simulink model "cddDcmApp"  (R2022b)
settings: Solver=FixedStepDiscrete  StartTime=0.0  StopTime=10.0  FixedStep=0.02  RelTol=1e-3
undefined symbols (not defined in the file or in its folder): CCPFaultType, DcChlType, HFAChlType, IcuChannelType, MtrRoutStaEnum, P_SignalPartType, P_Std_ReturnType, PowerChlType, SrsAdChlType, VGAChlType
(part 2 of 13)

== system cddDcmApp/DataServices_DID_4756_DID_4756_Front_Mosfet_Blower_FB ==
  DataServices_DID_4756_DID_4756_Front_Mosfet_Blower_FB_Voltage_sys : Subsystem

== system cddDcmApp/DataServices_DID_47F3_DID_47F3_AIU_Failure_Reasons_and ==
  DataServices_DID_47F3_DID_47F3_AIU_Failure_Reasons_and_Status_sys : Subsystem

== system cddDcmApp/DataServices_DID_47F4_DID_47F4_AQS_Air_Quality ==
  DataServices_DID_47F4_DID_47F4_AQS_Air_Quality_Level_sys : Subsystem

== system cddDcmApp/DataServices_DID_47F5_DID_47F5_TestRunCriteria_TRC ==
  DataServices_DID_47F5_DID_47F5_TestRunCriteria_TRC_Result_sys : Subsystem

== system cddDcmApp/DataServices_DID_47F6_DID_47F6_CCM_Lin1_Frame ==
  DataServices_DID_47F6_DID_47F6_CCM_Lin1_Frame_Status_sys : Subsystem

== system cddDcmApp/DataServices_DID_47F7_DID_47F7_CCM_Lin2_Frame ==
  DataServices_DID_47F7_DID_47F7_CCM_Lin2_Frame_Status_sys : Subsystem

== system cddDcmApp/DataServices_DID_47F8_DID_47F8_CCM_Lin3_Frame ==
  DataServices_DID_47F8_DID_47F8_CCM_Lin3_Frame_Status_sys : Subsystem

== system cddDcmApp/DataServices_DID_47F9_DID_47F9_Blower_Max_Level_Voltage ==
  DataServices_DID_47F9_DID_47F9_Blower_Max_Level_Voltage_Limit_sys : Subsystem

== system cddDcmApp/DataServices_DID_4AF7_DID_4AF7_1_DC_Motor_Drive_Request_Number ==
  DataServices_DID_4AF7_DID_4AF7_1_DC_Motor_Drive_Request_Number_FreezeCurrentState_sys : Subsystem
  DataServices_DID_4AF7_DID_4AF7_1_DC_Motor_Drive_Request_Number_ReadData_sys : Subsystem
  DataServices_DID_4AF7_DID_4AF7_1_DC_Motor_Drive_Request_Number_ReturnControlToECU_sys : Subsystem
  DataServices_DID_4AF7_DID_4AF7_1_DC_Motor_Drive_Request_Number_ShortTermAdjustment_sys : Subsystem

== system cddDcmApp/DataServices_DID_4AF7_DID_4AF7_2_DC_Motor_Drive_Request_Time ==
  DataServices_DID_4AF7_DID_4AF7_2_DC_Motor_Drive_Request_Time_FreezeCurrentState_sys : Subsystem
  DataServices_DID_4AF7_DID_4AF7_2_DC_Motor_Drive_Request_Time_ReadData_sys : Subsystem
  DataServices_DID_4AF7_DID_4AF7_2_DC_Motor_Drive_Request_Time_ReturnControlToECU_sys : Subsystem
  DataServices_DID_4AF7_DID_4AF7_2_DC_Motor_Drive_Request_Time_ShortTermAdjustment_sys : Subsystem

== system cddDcmApp/DataServices_DID_4AF7_DID_4AF7_3_DC_Motor_Drive_Request_Direction ==
  DataServices_DID_4AF7_DID_4AF7_3_DC_Motor_Drive_Request_Direction_FreezeCurrentState_sys : Subsystem
  DataServices_DID_4AF7_DID_4AF7_3_DC_Motor_Drive_Request_Direction_ReadData_sys : Subsystem
  DataServices_DID_4AF7_DID_4AF7_3_DC_Motor_Drive_Request_Direction_ReturnControlToECU_sys : Subsystem
  DataServices_DID_4AF7_DID_4AF7_3_DC_Motor_Drive_Request_Direction_ShortTermAdjustment_sys : Subsystem

== system cddDcmApp/DataServices_DID_9800_DID_9800_1_Evaporator_Temperature ==
  DataServices_DID_9800_DID_9800_1_Evaporator_Temperature_ReadData_sys : Subsystem

== system cddDcmApp/DataServices_DID_9800_DID_9800_2_incar_temperature ==
  DataServices_DID_9800_DID_9800_2_incar_temperature_ReadData_sys : Subsystem

== system cddDcmApp/DataServices_DID_9800_DID_9800_3_outside_temperature ==
  DataServices_DID_9800_DID_9800_3_outside_temperature_ReadData_sys : Subsystem

== system cddDcmApp/DataServices_DID_9800_DID_9800_4_Hex_sensor ==
  DataServices_DID_9800_DID_9800_4_Hex_sensor_ReadData_sys : Subsystem

== system cddDcmApp/DataServices_DID_9801_DID_9801_1_Front_left_vent_temperature ==
  DataServices_DID_9801_DID_9801_1_Front_left_vent_temperature_ReadData_sys : Subsystem

== system cddDcmApp/DataServices_DID_9801_DID_9801_2_Front_left_floor_temperature ==
  DataServices_DID_9801_DID_9801_2_Front_left_floor_temperature_ReadData_sys : Subsystem

== system cddDcmApp/DataServices_DID_9801_DID_9801_3_Front_right_vent_temperature ==
  DataServices_DID_9801_DID_9801_3_Front_right_vent_temperature_ReadData_sys : Subsystem

== system cddDcmApp/DataServices_DID_9801_DID_9801_4_Front_right_floor_temperature ==
  DataServices_DID_9801_DID_9801_4_Front_right_floor_temperature_ReadData_sys : Subsystem

== system cddDcmApp/DataServices_DID_9802_1_Second_row_left_vent_temperatureSecond_row_vent_temperature ==
  DataServices_DID_9802_1_Second_row_left_vent_temperatureSecond_row_vent_temperature_ReadData_sys : Subsystem

== system cddDcmApp/DataServices_DID_9802_2_Second_row_left_floor_temperatureSecond_row_floor_temperature ==
  DataServices_DID_9802_2_Second_row_left_floor_temperatureSecond_row_floor_temperature_ReadData_sys : Subsystem

== system cddDcmApp/DataServices_DID_9802_DID_9802_3_Second_row_right_vent_temperature ==
  DataServices_DID_9802_DID_9802_3_Second_row_right_vent_temperature_ReadData_sys : Subsystem

== system cddDcmApp/DataServices_DID_9802_DID_9802_4_Second_row_right_floor_temperature ==
  DataServices_DID_9802_DID_9802_4_Second_row_right_floor_temperature_ReadData_sys : Subsystem

== system cddDcmApp/DataServices_DID_9803_DID_9803_1_Left_sun_intensity ==
  DataServices_DID_9803_DID_9803_1_Left_sun_intensity_ReadData_sys : Subsystem

== system cddDcmApp/DataServices_DID_9803_DID_9803_2_Right_sun_intensity ==
  DataServices_DID_9803_DID_9803_2_Right_sun_intensity_ReadData_sys : Subsystem

== system cddDcmApp/DataServices_DID_9803_DID_9803_3_engine_coolant_temperature ==
  DataServices_DID_9803_DID_9803_3_engine_coolant_temperature_ReadData_sys : Subsystem

== system cddDcmApp/DataServices_DID_9803_DID_9803_4_dew_temperature ==
  DataServices_DID_9803_DID_9803_4_dew_temperature_ReadData_sys : Subsystem

== system cddDcmApp/DataServices_DID_9804_DID_9804_1_Relative_Humidity ==
  DataServices_DID_9804_DID_9804_1_Relative_Humidity_ReadData_sys : Subsystem

== system cddDcmApp/DataServices_DID_9804_DID_9804_2_AQS_sensor ==
  DataServices_DID_9804_DID_9804_2_AQS_sensor_ReadData_sys : Subsystem

== system cddDcmApp/DataServices_DID_9804_DID_9804_3_PM25PM25_in_sensor_concentration ==
  DataServices_DID_9804_DID_9804_3_PM25PM25_in_sensor_concentration_ReadData_sys : Subsystem

== system cddDcmApp/DataServices_DID_9804_DID_9804_4_PM25_out_sensor_concentration ==
  DataServices_DID_9804_DID_9804_4_PM25_out_sensor_concentration_ReadData_sys : Subsystem

== system cddDcmApp/DataServices_DID_9805_DID_9805_1_CO2_sensor_concentration ==
  DataServices_DID_9805_DID_9805_1_CO2_sensor_concentration_ReadData_sys : Subsystem

== system cddDcmApp/DataServices_DID_9805_DID_9805_2_Front_windscreen_temperature ==
  DataServices_DID_9805_DID_9805_2_Front_windscreen_temperature_ReadData_sys : Subsystem

== system cddDcmApp/DataServices_DID_9805_DID_9805_3_Rear_cabin_temperature ==
  DataServices_DID_9805_DID_9805_3_Rear_cabin_temperature_ReadData_sys : Subsystem

== system cddDcmApp/DataServices_DID_9805_DID_9805_4_Third_row_air_temperature ==
  DataServices_DID_9805_DID_9805_4_Third_row_air_temperature_ReadData_sys : Subsystem

== system cddDcmApp/DataServices_DID_9808_DID_9808_RHFA_Actuator_Position ==
  DataServices_DID_9808_DID_9808_RHFA_Actuator_Position_FreezeCurrentState_sys : Subsystem
  DataServices_DID_9808_DID_9808_RHFA_Actuator_Position_ReadData_sys : Subsystem
  DataServices_DID_9808_DID_9808_RHFA_Actuator_Position_ReturnControlToECU_sys : Subsystem
  DataServices_DID_9808_DID_9808_RHFA_Actuator_Position_ShortTermAdjustment_sys : Subsystem

== system cddDcmApp/DataServices_DID_9809_3_actuator_positionSecond_temperature_actuator_position ==
  DataServices_DID_9809_3_actuator_positionSecond_temperature_actuator_position_FreezeCurrentState_sys : Subsystem
  DataServices_DID_9809_3_actuator_positionSecond_temperature_actuator_position_ReadData_sys : Subsystem
  DataServices_DID_9809_3_actuator_positionSecond_temperature_actuator_position_ReturnControlToECU_sys : Subsystem
  DataServices_DID_9809_3_actuator_positionSecond_temperature_actuator_position_ShortTermAdjustment_sys : Subsystem

== system cddDcmApp/DataServices_DID_9809_DID_9809_1_Front_left_temperature_actuator_position ==
  DataServices_DID_9809_DID_9809_1_Front_left_temperature_actuator_position_FreezeCurrentState_sys : Subsystem
  DataServices_DID_9809_DID_9809_1_Front_left_temperature_actuator_position_ReadData_sys : Subsystem
  DataServices_DID_9809_DID_9809_1_Front_left_temperature_actuator_position_ReturnControlToECU_sys : Subsystem
  DataServices_DID_9809_DID_9809_1_Front_left_temperature_actuator_position_ShortTermAdjustment_sys : Subsystem

== system cddDcmApp/DataServices_DID_9809_DID_9809_2_Front_right_temperature_actuator_position ==
  DataServices_DID_9809_DID_9809_2_Front_right_temperature_actuator_position_FreezeCurrentState_sys : Subsystem
  DataServices_DID_9809_DID_9809_2_Front_right_temperature_actuator_position_ReadData_sys : Subsystem
  DataServices_DID_9809_DID_9809_2_Front_right_temperature_actuator_position_ReturnControlToECU_sys : Subsystem
  DataServices_DID_9809_DID_9809_2_Front_right_temperature_actuator_position_ShortTermAdjustment_sys : Subsystem

== system cddDcmApp/DataServices_DID_9809_DID_9809_4_Second_right_temperature_actuator_position ==
  DataServices_DID_9809_DID_9809_4_Second_right_temperature_actuator_position_FreezeCurrentState_sys : Subsystem
  DataServices_DID_9809_DID_9809_4_Second_right_temperature_actuator_position_ReadData_sys : Subsystem
  DataServices_DID_9809_DID_9809_4_Second_right_temperature_actuator_position_ReturnControlToECU_sys : Subsystem
  DataServices_DID_9809_DID_9809_4_Second_right_temperature_actuator_position_ShortTermAdjustment_sys : Subsystem

== system cddDcmApp/DataServices_DID_980A_2_Front_ventfloor_actuator_positionfront_vent_actuator_position ==
  DataServices_DID_980A_2_Front_ventfloor_actuator_positionfront_vent_actuator_position_FreezeCurrentState_sys : Subsystem
  DataServices_DID_980A_2_Front_ventfloor_actuator_positionfront_vent_actuator_position_ReadData_sys : Subsystem
  DataServices_DID_980A_2_Front_ventfloor_actuator_positionfront_vent_actuator_position_ReturnControlToECU_sys : Subsystem
  DataServices_DID_980A_2_Front_ventfloor_actuator_positionfront_vent_actuator_position_ShortTermAdjustment_sys : Subsystem

== system cddDcmApp/DataServices_DID_980A_DID_980A_1_Deforst_actuator_position ==
  DataServices_DID_980A_DID_980A_1_Deforst_actuator_position_FreezeCurrentState_sys : Subsystem
  DataServices_DID_980A_DID_980A_1_Deforst_actuator_position_ReadData_sys : Subsystem
  DataServices_DID_980A_DID_980A_1_Deforst_actuator_position_ReturnControlToECU_sys : Subsystem
  DataServices_DID_980A_DID_980A_1_Deforst_actuator_position_ShortTermAdjustment_sys : Subsystem

== system cddDcmApp/DataServices_DID_980A_DID_980A_3_Front_floor_actuator_positionposition ==
  DataServices_DID_980A_DID_980A_3_Front_floor_actuator_positionposition_FreezeCurrentState_sys : Subsystem
  DataServices_DID_980A_DID_980A_3_Front_floor_actuator_positionposition_ReadData_sys : Subsystem
  DataServices_DID_980A_DID_980A_3_Front_floor_actuator_positionposition_ReturnControlToECU_sys : Subsystem
  DataServices_DID_980A_DID_980A_3_Front_floor_actuator_positionposition_ShortTermAdjustment_sys : Subsystem

== system cddDcmApp/DataServices_DID_980A_DID_980A_4_Second_row_ventventfloor_actuator_position ==
  DataServices_DID_980A_DID_980A_4_Second_row_ventventfloor_actuator_position_FreezeCurrentState_sys : Subsystem
  DataServices_DID_980A_DID_980A_4_Second_row_ventventfloor_actuator_position_ReadData_sys : Subsystem
  DataServices_DID_980A_DID_980A_4_Second_row_ventventfloor_actuator_position_ReturnControlToECU_sys : Subsystem
  DataServices_DID_980A_DID_980A_4_Second_row_ventventfloor_actuator_position_ShortTermAdjustment_sys : Subsystem

== system cddDcmApp/DataServices_DID_980B_DID_980B_10_VGA7Passenger_Right_X_actuator_positon ==
  DataServices_DID_980B_DID_980B_10_VGA7Passenger_Right_X_actuator_positon_FreezeCurrentState_sys : Subsystem
  DataServices_DID_980B_DID_980B_10_VGA7Passenger_Right_X_actuator_positon_ReadData_sys : Subsystem
  DataServices_DID_980B_DID_980B_10_VGA7Passenger_Right_X_actuator_positon_ReturnControlToECU_sys : Subsystem
  DataServices_DID_980B_DID_980B_10_VGA7Passenger_Right_X_actuator_positon_ShortTermAdjustment_sys : Subsystem

== system cddDcmApp/DataServices_DID_980B_DID_980B_11_VGA8Passenger_Right_Y_actuator_positon ==
  DataServices_DID_980B_DID_980B_11_VGA8Passenger_Right_Y_actuator_positon_FreezeCurrentState_sys : Subsystem
  DataServices_DID_980B_DID_980B_11_VGA8Passenger_Right_Y_actuator_positon_ReadData_sys : Subsystem
  DataServices_DID_980B_DID_980B_11_VGA8Passenger_Right_Y_actuator_positon_ReturnControlToECU_sys : Subsystem
  DataServices_DID_980B_DID_980B_11_VGA8Passenger_Right_Y_actuator_positon_ShortTermAdjustment_sys : Subsystem

== system cddDcmApp/DataServices_DID_980B_DID_980B_1_second_row_floor_actuator_position ==
  DataServices_DID_980B_DID_980B_1_second_row_floor_actuator_position_FreezeCurrentState_sys : Subsystem
  DataServices_DID_980B_DID_980B_1_second_row_floor_actuator_position_ReadData_sys : Subsystem
  DataServices_DID_980B_DID_980B_1_second_row_floor_actuator_position_ReturnControlToECU_sys : Subsystem
  DataServices_DID_980B_DID_980B_1_second_row_floor_actuator_position_ShortTermAdjustment_sys : Subsystem

== system cddDcmApp/DataServices_DID_980B_DID_980B_2_Rec_actuator_position ==
  DataServices_DID_980B_DID_980B_2_Rec_actuator_position_FreezeCurrentState_sys : Subsystem
  DataServices_DID_980B_DID_980B_2_Rec_actuator_position_ReadData_sys : Subsystem
  DataServices_DID_980B_DID_980B_2_Rec_actuator_position_ReturnControlToECU_sys : Subsystem
  DataServices_DID_980B_DID_980B_2_Rec_actuator_position_ShortTermAdjustment_sys : Subsystem

== system cddDcmApp/DataServices_DID_980B_DID_980B_3_Osa_actuator_position ==
  DataServices_DID_980B_DID_980B_3_Osa_actuator_position_FreezeCurrentState_sys : Subsystem
  DataServices_DID_980B_DID_980B_3_Osa_actuator_position_ReadData_sys : Subsystem
  DataServices_DID_980B_DID_980B_3_Osa_actuator_position_ReturnControlToECU_sys : Subsystem
  DataServices_DID_980B_DID_980B_3_Osa_actuator_position_ShortTermAdjustment_sys : Subsystem

== system cddDcmApp/DataServices_DID_980B_DID_980B_4_VGA1Driver_Left_X_actuator_positon ==
  DataServices_DID_980B_DID_980B_4_VGA1Driver_Left_X_actuator_positon_FreezeCurrentState_sys : Subsystem
  DataServices_DID_980B_DID_980B_4_VGA1Driver_Left_X_actuator_positon_ReadData_sys : Subsystem
  DataServices_DID_980B_DID_980B_4_VGA1Driver_Left_X_actuator_positon_ReturnControlToECU_sys : Subsystem
  DataServices_DID_980B_DID_980B_4_VGA1Driver_Left_X_actuator_positon_ShortTermAdjustment_sys : Subsystem

== system cddDcmApp/DataServices_DID_980B_DID_980B_5_VGA2Driver_Left_Y_actuator_positon ==
  DataServices_DID_980B_DID_980B_5_VGA2Driver_Left_Y_actuator_positon_FreezeCurrentState_sys : Subsystem
  DataServices_DID_980B_DID_980B_5_VGA2Driver_Left_Y_actuator_positon_ReadData_sys : Subsystem
  DataServices_DID_980B_DID_980B_5_VGA2Driver_Left_Y_actuator_positon_ReturnControlToECU_sys : Subsystem
  DataServices_DID_980B_DID_980B_5_VGA2Driver_Left_Y_actuator_positon_ShortTermAdjustment_sys : Subsystem

== system cddDcmApp/DataServices_DID_980B_DID_980B_6_VGA3Driver_Right_X_actuator_positon ==
  DataServices_DID_980B_DID_980B_6_VGA3Driver_Right_X_actuator_positon_FreezeCurrentState_sys : Subsystem
  DataServices_DID_980B_DID_980B_6_VGA3Driver_Right_X_actuator_positon_ReadData_sys : Subsystem
  DataServices_DID_980B_DID_980B_6_VGA3Driver_Right_X_actuator_positon_ReturnControlToECU_sys : Subsystem
  DataServices_DID_980B_DID_980B_6_VGA3Driver_Right_X_actuator_positon_ShortTermAdjustment_sys : Subsystem

== system cddDcmApp/DataServices_DID_980B_DID_980B_7_VGA4Driver_Right_Y_actuator_positon ==
  DataServices_DID_980B_DID_980B_7_VGA4Driver_Right_Y_actuator_positon_FreezeCurrentState_sys : Subsystem
  DataServices_DID_980B_DID_980B_7_VGA4Driver_Right_Y_actuator_positon_ReadData_sys : Subsystem
  DataServices_DID_980B_DID_980B_7_VGA4Driver_Right_Y_actuator_positon_ReturnControlToECU_sys : Subsystem
  DataServices_DID_980B_DID_980B_7_VGA4Driver_Right_Y_actuator_positon_ShortTermAdjustment_sys : Subsystem

== system cddDcmApp/DataServices_DID_980B_DID_980B_8_VGA5Passenger_Left_X_actuator_positon ==
  DataServices_DID_980B_DID_980B_8_VGA5Passenger_Left_X_actuator_positon_FreezeCurrentState_sys : Subsystem
  DataServices_DID_980B_DID_980B_8_VGA5Passenger_Left_X_actuator_positon_ReadData_sys : Subsystem
  DataServices_DID_980B_DID_980B_8_VGA5Passenger_Left_X_actuator_positon_ReturnControlToECU_sys : Subsystem
  DataServices_DID_980B_DID_980B_8_VGA5Passenger_Left_X_actuator_positon_ShortTermAdjustment_sys : Subsystem

== system cddDcmApp/DataServices_DID_980B_DID_980B_9_VGA6Passenger_Left_Y_actuator_positon ==
  DataServices_DID_980B_DID_980B_9_VGA6Passenger_Left_Y_actuator_positon_FreezeCurrentState_sys : Subsystem
  DataServices_DID_980B_DID_980B_9_VGA6Passenger_Left_Y_actuator_positon_ReadData_sys : Subsystem
  DataServices_DID_980B_DID_980B_9_VGA6Passenger_Left_Y_actuator_positon_ReturnControlToECU_sys : Subsystem
  DataServices_DID_980B_DID_980B_9_VGA6Passenger_Left_Y_actuator_positon_ShortTermAdjustment_sys : Subsystem

== system cddDcmApp/DataServices_DID_980E_DID_980E_1_Front_Blower_pwm ==
  DataServices_DID_980E_DID_980E_1_Front_Blower_pwm_FreezeCurrentState_sys : Subsystem
  DataServices_DID_980E_DID_980E_1_Front_Blower_pwm_ReadData_sys : Subsystem
  DataServices_DID_980E_DID_980E_1_Front_Blower_pwm_ReturnControlToECU_sys : Subsystem
  DataServices_DID_980E_DID_980E_1_Front_Blower_pwm_ShortTermAdjustment_sys : Subsystem

== system cddDcmApp/DataServices_DID_980E_DID_980E_2_Front_Blower_speedLIN1 ==
  DataServices_DID_980E_DID_980E_2_Front_Blower_speedLIN1_FreezeCurrentState_sys : Subsystem
  DataServices_DID_980E_DID_980E_2_Front_Blower_speedLIN1_ReadData_sys : Subsystem
  DataServices_DID_980E_DID_980E_2_Front_Blower_speedLIN1_ReturnControlToECU_sys : Subsystem
  DataServices_DID_980E_DID_980E_2_Front_Blower_speedLIN1_ShortTermAdjustment_sys : Subsystem

== system cddDcmApp/DataServices_DID_980E_DID_980E_3_Incar_motorActvCmd ==
  DataServices_DID_980E_DID_980E_3_Incar_motorActvCmd_FreezeCurrentState_sys : Subsystem
  DataServices_DID_980E_DID_980E_3_Incar_motorActvCmd_ReadData_sys : Subsystem
  DataServices_DID_980E_DID_980E_3_Incar_motorActvCmd_ReturnControlToECU_sys : Subsystem
  DataServices_DID_980E_DID_980E_3_Incar_motorActvCmd_ShortTermAdjustment_sys : Subsystem

== system cddDcmApp/DataServices_DID_980E_DID_980E_4_Rear_blower_speed ==
  DataServices_DID_980E_DID_980E_4_Rear_blower_speed_FreezeCurrentState_sys : Subsystem
  DataServices_DID_980E_DID_980E_4_Rear_blower_speed_ReadData_sys : Subsystem
  DataServices_DID_980E_DID_980E_4_Rear_blower_speed_ReturnControlToECU_sys : Subsystem
  DataServices_DID_980E_DID_980E_4_Rear_blower_speed_ShortTermAdjustment_sys : Subsystem

== system cddDcmApp/DataServices_DID_980F_Data_Record ==
  DataServices_DID_980F_Data_Record_FreezeCurrentState_sys : Subsystem
  DataServices_DID_980F_Data_Record_ReadData_sys : Subsystem
  DataServices_DID_980F_Data_Record_ReturnControlToECU_sys : Subsystem
  DataServices_DID_980F_Data_Record_ShortTermAdjustment_sys : Subsystem

== system cddDcmApp/DataServices_DID_987B_DID_987B_HBMF_Failure ==
  DataServices_DID_987B_DID_987B_HBMF_Failure_Reasons_sys : Subsystem

== system cddDcmApp/DataServices_DID_987C_DID_987C_HBMF_Feedback ==
  DataServices_DID_987C_DID_987C_HBMF_Feedback_Datalog_sys : Subsystem

== system cddDcmApp/DataServices_DID_987D_DID_987D_PMSI_Failure ==
  DataServices_DID_987D_DID_987D_PMSI_Failure_Reasons_sys : Subsystem

== system cddDcmApp/DataServices_DID_987E_DID_987E_AFU_Failure ==
  DataServices_DID_987E_DID_987E_AFU_Failure_Reasons_sys : Subsystem

== system cddDcmApp/DataServices_DID_987F_DID_987F_CCM_Low_Voltage_Power_Limited ==
  DataServices_DID_987F_DID_987F_CCM_Low_Voltage_Power_Limited_Infor_sys : Subsystem

== system cddDcmApp/DataServices_DID_9880_DID_9880_PWM_Blower_Error_Internal_Info ==
  DataServices_DID_9880_DID_9880_PWM_Blower_Error_Internal_Info_Datalog_sys : Subsystem

== system cddDcmApp/DataServices_DID_988E_DID_988E_Front_PWM_Blower_Feedback ==
  DataServices_DID_988E_DID_988E_Front_PWM_Blower_Feedback_Datalog_sys : Subsystem

== system cddDcmApp/DataServices_DID_988F_DID_988F_Rear_Blower_Feedback ==
  DataServices_DID_988F_DID_988F_Rear_Blower_Feedback_Voltage_sys : Subsystem

== system cddDcmApp/DataServices_DID_9890_DID_9890_VGA1_Failure ==
  DataServices_DID_9890_DID_9890_VGA1_Failure_Reasons_sys : Subsystem

== system cddDcmApp/DataServices_DID_9891_DID_9891_VGA2_Failure ==
  DataServices_DID_9891_DID_9891_VGA2_Failure_Reasons_sys : Subsystem

== system cddDcmApp/DataServices_DID_9892_DID_9892_VGA3_Failure ==
  DataServices_DID_9892_DID_9892_VGA3_Failure_Reasons_sys : Subsystem

== system cddDcmApp/DataServices_DID_9893_DID_9893_VGA4_Failure ==
  DataServices_DID_9893_DID_9893_VGA4_Failure_Reasons_sys : Subsystem

== system cddDcmApp/DataServices_DID_9894_DID_9894_VGA5_Failure ==
  DataServices_DID_9894_DID_9894_VGA5_Failure_Reasons_sys : Subsystem

== system cddDcmApp/DataServices_DID_9895_DID_9895_VGA6_Failure ==
  DataServices_DID_9895_DID_9895_VGA6_Failure_Reasons_sys : Subsystem

== system cddDcmApp/DataServices_DID_9896_DID_9896_VGA7_Failure ==
  DataServices_DID_9896_DID_9896_VGA7_Failure_Reasons_sys : Subsystem

== system cddDcmApp/DataServices_DID_9897_DID_9897_VGA8_Failure ==
  DataServices_DID_9897_DID_9897_VGA8_Failure_Reasons_sys : Subsystem

== system cddDcmApp/DataServices_DID_9898_DID_9898_Sensor_AD ==
  DataServices_DID_9898_DID_9898_Sensor_AD_Value_sys : Subsystem

== system cddDcmApp/DataServices_DID_989C_DID_989C_Rear_LIN_Blower_Module_VBMR_Fan_Status ==
  DataServices_DID_989C_DID_989C_Rear_LIN_Blower_Module_VBMR_Fan_Status_ReadData_sys : Subsystem

== system cddDcmApp/DataServices_DID_989D_DID_989D_Rear_LIN_Blower_Module_VBMR_Feedback_Datalog ==
  DataServices_DID_989D_DID_989D_Rear_LIN_Blower_Module_VBMR_Feedback_Datalog_ReadData_sys : Subsystem

== system cddDcmApp/DataServices_DID_989E_DID_989E_Rear_HVAC_Flap_Actuator_RHFA_Failure_Reasons ==
  DataServices_DID_989E_DID_989E_Rear_HVAC_Flap_Actuator_RHFA_Failure_Reasons_ReadData_sys : Subsystem

== system cddDcmApp/DataServices_DID_989F_DID_989F_CCM_LIN_Actuator_Frame_Status ==
  DataServices_DID_989F_DID_989F_CCM_LIN_Actuator_Frame_Status_ReadData_sys : Subsystem

== system cddDcmApp/DataServices_DID_98A1_DID_98A1_98A1_DC_Motor_Feedback_Voltage ==
  DataServices_DID_98A1_DID_98A1_98A1_DC_Motor_Feedback_Voltage_ReadData_sys : Subsystem

== system cddDcmApp/DataServices_DID_98A2_DID_98A2_Front_Lpm_Blower_Feedback_Voltage_Difference ==
  DataServices_DID_98A2_DID_98A2_Front_Lpm_Blower_Feedback_Voltage_Difference_ReadData_sys : Subsystem

== system cddDcmApp/DataServices_DID_98A3_DID_98A3_Advanced_Air_Cleaner_AAC_Feedback_Info ==
  DataServices_DID_98A3_DID_98A3_Advanced_Air_Cleaner_AAC_Feedback_Info_ReadData_sys : Subsystem

== system cddDcmApp/DataServices_DID_98A4_DID_98A4_Oxygen_Generator_Module_OGM_Feedback_Info ==
  DataServices_DID_98A4_DID_98A4_Oxygen_Generator_Module_OGM_Feedback_Info_ReadData_sys : Subsystem

== system cddDcmApp/DataServices_DID_98A5_DID_98A5_Interior_Oxygen_Concentration_Sensor_IOSM_Module_Feedback_Info ==
  DataServices_DID_98A5_DID_98A5_Interior_Oxygen_Concentration_Sensor_IOSM_Module_Feedback_Info_ReadData_sys : Subsystem

== system cddDcmApp/DataServices_DID_D910_DID_D910_BLW_THM_Output ==
  DataServices_DID_D910_DID_D910_BLW_THM_Output_ReadData_sys : Subsystem

== system cddDcmApp/DataServices_DID_D922_DID_D922_1_HVAC_Front_Blower_control_level ==
  DataServices_DID_D922_DID_D922_1_HVAC_Front_Blower_control_level_ReadData_sys : Subsystem

== system cddDcmApp/DataServices_DID_D922_DID_D922_2_HVAC_Rear_Blower_control_level ==
  DataServices_DID_D922_DID_D922_2_HVAC_Rear_Blower_control_level_ReadData_sys : Subsystem

== system cddDcmApp/DataServices_DID_D924_DID_D924_1_No_Of_Movements ==
  DataServices_DID_D924_DID_D924_1_No_Of_Movements_ReadData_sys : Subsystem
  DataServices_DID_D924_DID_D924_1_No_Of_Movements_WriteData_sys : Subsystem

== system cddDcmApp/DataServices_DID_D924_DID_D924_2_ADC_value_Lower_Endstop_at_0 ==
  DataServices_DID_D924_DID_D924_2_ADC_value_Lower_Endstop_at_0_ReadData_sys : Subsystem
  DataServices_DID_D924_DID_D924_2_ADC_value_Lower_Endstop_at_0_WriteData_sys : Subsystem

== system cddDcmApp/DataServices_DID_D924_DID_D924_3_ADC_value_Upper_Endstop_at_100 ==
  DataServices_DID_D924_DID_D924_3_ADC_value_Upper_Endstop_at_100_ReadData_sys : Subsystem
  DataServices_DID_D924_DID_D924_3_ADC_value_Upper_Endstop_at_100_WriteData_sys : Subsystem

== system cddDcmApp/DataServices_DID_D925_ComplexStructure_2 ==
  DataServices_DID_D925_ComplexStructure_2_ReadData_sys : Subsystem

== system cddDcmApp/DataServices_DID_D925_DID_D925_1_Requested_Position ==
  DataServices_DID_D925_DID_D925_1_Requested_Position_ReadData_sys : Subsystem

== system cddDcmApp/DataServices_DID_D925_DID_D925_2_Actual_Position ==
  DataServices_DID_D925_DID_D925_2_Actual_Position_ReadData_sys : Subsystem

== system cddDcmApp/DataServices_DID_D926_DID_D926_1_No_Of_Movements ==
  DataServices_DID_D926_DID_D926_1_No_Of_Movements_ReadData_sys : Subsystem
  DataServices_DID_D926_DID_D926_1_No_Of_Movements_WriteData_sys : Subsystem

== system cddDcmApp/DataServices_DID_D926_DID_D926_2_ADC_value_Lower_Endstop_at_0 ==
  DataServices_DID_D926_DID_D926_2_ADC_value_Lower_Endstop_at_0_ReadData_sys : Subsystem
  DataServices_DID_D926_DID_D926_2_ADC_value_Lower_Endstop_at_0_WriteData_sys : Subsystem

== system cddDcmApp/DataServices_DID_D926_DID_D926_3_ADC_value_Upper_Endstop_at_100 ==
  DataServices_DID_D926_DID_D926_3_ADC_value_Upper_Endstop_at_100_ReadData_sys : Subsystem
  DataServices_DID_D926_DID_D926_3_ADC_value_Upper_Endstop_at_100_WriteData_sys : Subsystem

== system cddDcmApp/DataServices_DID_D927_ComplexStructure_2 ==
  DataServices_DID_D927_ComplexStructure_2_ReadData_sys : Subsystem

== system cddDcmApp/DataServices_DID_D927_DID_D927_1_Requested_Position ==
  DataServices_DID_D927_DID_D927_1_Requested_Position_ReadData_sys : Subsystem

== system cddDcmApp/DataServices_DID_D927_DID_D927_2_Actual_Position ==
  DataServices_DID_D927_DID_D927_2_Actual_Position_ReadData_sys : Subsystem

== system cddDcmApp/DataServices_DID_D928_DID_D928_1_No_Of_Movements ==
  DataServices_DID_D928_DID_D928_1_No_Of_Movements_ReadData_sys : Subsystem
  DataServices_DID_D928_DID_D928_1_No_Of_Movements_WriteData_sys : Subsystem

== system cddDcmApp/DataServices_DID_D928_DID_D928_2_ADC_value_Lower_Endstop_at_0 ==
  DataServices_DID_D928_DID_D928_2_ADC_value_Lower_Endstop_at_0_ReadData_sys : Subsystem
  DataServices_DID_D928_DID_D928_2_ADC_value_Lower_Endstop_at_0_WriteData_sys : Subsystem

== system cddDcmApp/DataServices_DID_D928_DID_D928_3_ADC_value_Upper_Endstop_at_100 ==
  DataServices_DID_D928_DID_D928_3_ADC_value_Upper_Endstop_at_100_ReadData_sys : Subsystem
  DataServices_DID_D928_DID_D928_3_ADC_value_Upper_Endstop_at_100_WriteData_sys : Subsystem

== system cddDcmApp/DataServices_DID_D929_ComplexStructure_2 ==
  DataServices_DID_D929_ComplexStructure_2_ReadData_sys : Subsystem

== system cddDcmApp/DataServices_DID_D929_DID_D929_1_Requested_Position ==
  DataServices_DID_D929_DID_D929_1_Requested_Position_ReadData_sys : Subsystem

== system cddDcmApp/DataServices_DID_D929_DID_D929_2_Actual_Position ==
  DataServices_DID_D929_DID_D929_2_Actual_Position_ReadData_sys : Subsystem

== system cddDcmApp/DataServices_DID_D92A_DID_D92A_1_No_Of_Movements ==
  DataServices_DID_D92A_DID_D92A_1_No_Of_Movements_ReadData_sys : Subsystem
  DataServices_DID_D92A_DID_D92A_1_No_Of_Movements_WriteData_sys : Subsystem

== system cddDcmApp/DataServices_DID_D92A_DID_D92A_2_ADC_value_Lower_Endstop_at_0 ==
  DataServices_DID_D92A_DID_D92A_2_ADC_value_Lower_Endstop_at_0_ReadData_sys : Subsystem
  DataServices_DID_D92A_DID_D92A_2_ADC_value_Lower_Endstop_at_0_WriteData_sys : Subsystem

== system cddDcmApp/DataServices_DID_D92A_DID_D92A_3_ADC_value_Upper_Endstop_at_100 ==
  DataServices_DID_D92A_DID_D92A_3_ADC_value_Upper_Endstop_at_100_ReadData_sys : Subsystem
  DataServices_DID_D92A_DID_D92A_3_ADC_value_Upper_Endstop_at_100_WriteData_sys : Subsystem

== system cddDcmApp/DataServices_DID_D92B_ComplexStructure_2 ==
  DataServices_DID_D92B_ComplexStructure_2_ReadData_sys : Subsystem

== system cddDcmApp/DataServices_DID_D92B_DID_D92B_1_Requested_Position ==
  DataServices_DID_D92B_DID_D92B_1_Requested_Position_ReadData_sys : Subsystem

== system cddDcmApp/DataServices_DID_D92B_DID_D92B_2_Actual_Position ==
  DataServices_DID_D92B_DID_D92B_2_Actual_Position_ReadData_sys : Subsystem

== system cddDcmApp/DataServices_DID_D92C_DID_D92C_1_No_Of_Movements ==
  DataServices_DID_D92C_DID_D92C_1_No_Of_Movements_ReadData_sys : Subsystem
  DataServices_DID_D92C_DID_D92C_1_No_Of_Movements_WriteData_sys : Subsystem

== system cddDcmApp/DataServices_DID_D92C_DID_D92C_2_ADC_value_Lower_Endstop_at_0 ==
  DataServices_DID_D92C_DID_D92C_2_ADC_value_Lower_Endstop_at_0_ReadData_sys : Subsystem
  DataServices_DID_D92C_DID_D92C_2_ADC_value_Lower_Endstop_at_0_WriteData_sys : Subsystem

== system cddDcmApp/DataServices_DID_D92C_DID_D92C_3_ADC_value_Upper_Endstop_at_100 ==
  DataServices_DID_D92C_DID_D92C_3_ADC_value_Upper_Endstop_at_100_ReadData_sys : Subsystem
  DataServices_DID_D92C_DID_D92C_3_ADC_value_Upper_Endstop_at_100_WriteData_sys : Subsystem

== system cddDcmApp/DataServices_DID_D92D_ComplexStructure_2 ==
  DataServices_DID_D92D_ComplexStructure_2_ReadData_sys : Subsystem

== system cddDcmApp/DataServices_DID_D92D_DID_D92D_1_Requested_Position ==
  DataServices_DID_D92D_DID_D92D_1_Requested_Position_ReadData_sys : Subsystem

== system cddDcmApp/DataServices_DID_D92D_DID_D92D_2_Actual_Position ==
  DataServices_DID_D92D_DID_D92D_2_Actual_Position_ReadData_sys : Subsystem

== system cddDcmApp/DataServices_DID_D92E_DID_D92E_1_No_Of_Movements ==
  DataServices_DID_D92E_DID_D92E_1_No_Of_Movements_ReadData_sys : Subsystem
  DataServices_DID_D92E_DID_D92E_1_No_Of_Movements_WriteData_sys : Subsystem

== system cddDcmApp/DataServices_DID_D92E_DID_D92E_2_ADC_value_Lower_Endstop_at_0 ==
  DataServices_DID_D92E_DID_D92E_2_ADC_value_Lower_Endstop_at_0_ReadData_sys : Subsystem
  DataServices_DID_D92E_DID_D92E_2_ADC_value_Lower_Endstop_at_0_WriteData_sys : Subsystem

== system cddDcmApp/DataServices_DID_D92E_DID_D92E_3_ADC_value_Upper_Endstop_at_100 ==
  DataServices_DID_D92E_DID_D92E_3_ADC_value_Upper_Endstop_at_100_ReadData_sys : Subsystem
  DataServices_DID_D92E_DID_D92E_3_ADC_value_Upper_Endstop_at_100_WriteData_sys : Subsystem

== system cddDcmApp/DataServices_DID_D92F_ComplexStructure_2 ==
  DataServices_DID_D92F_ComplexStructure_2_ReadData_sys : Subsystem

== system cddDcmApp/DataServices_DID_D92F_DID_D92F_1_Requested_Position ==
  DataServices_DID_D92F_DID_D92F_1_Requested_Position_ReadData_sys : Subsystem

== system cddDcmApp/DataServices_DID_D92F_DID_D92F_2_Actual_Position ==
  DataServices_DID_D92F_DID_D92F_2_Actual_Position_ReadData_sys : Subsystem

== system cddDcmApp/DataServices_DID_D930_DID_D930_1_No_Of_Movements ==
  DataServices_DID_D930_DID_D930_1_No_Of_Movements_ReadData_sys : Subsystem
  DataServices_DID_D930_DID_D930_1_No_Of_Movements_WriteData_sys : Subsystem

== system cddDcmApp/DataServices_DID_D930_DID_D930_2_ADC_value_Lower_Endstop_at_0 ==
  DataServices_DID_D930_DID_D930_2_ADC_value_Lower_Endstop_at_0_ReadData_sys : Subsystem
  DataServices_DID_D930_DID_D930_2_ADC_value_Lower_Endstop_at_0_WriteData_sys : Subsystem

== system cddDcmApp/DataServices_DID_D930_DID_D930_3_ADC_value_Upper_Endstop_at_100 ==
  DataServices_DID_D930_DID_D930_3_ADC_value_Upper_Endstop_at_100_ReadData_sys : Subsystem
  DataServices_DID_D930_DID_D930_3_ADC_value_Upper_Endstop_at_100_WriteData_sys : Subsystem

== system cddDcmApp/DataServices_DID_D931_ComplexStructure_2 ==
  DataServices_DID_D931_ComplexStructure_2_ReadData_sys : Subsystem

== system cddDcmApp/DataServices_DID_D931_DID_D931_1_Requested_Position ==
  DataServices_DID_D931_DID_D931_1_Requested_Position_ReadData_sys : Subsystem

== system cddDcmApp/DataServices_DID_D931_DID_D931_2_Actual_Position ==
  DataServices_DID_D931_DID_D931_2_Actual_Position_ReadData_sys : Subsystem

== system cddDcmApp/DataServices_DID_D933_DID_D933_1_step_Range ==
  DataServices_DID_D933_DID_D933_1_step_Range_ReadData_sys : Subsystem
  DataServices_DID_D933_DID_D933_1_step_Range_WriteData_sys : Subsystem

== system cddDcmApp/DataServices_DID_D933_DID_D933_2_No_Of_Movements ==
  DataServices_DID_D933_DID_D933_2_No_Of_Movements_ReadData_sys : Subsystem
  DataServices_DID_D933_DID_D933_2_No_Of_Movements_WriteData_sys : Subsystem

== system cddDcmApp/DataServices_DID_D934_ComplexStructure_2 ==
  DataServices_DID_D934_ComplexStructure_2_ReadData_sys : Subsystem

== system cddDcmApp/DataServices_DID_D934_DID_D934_1_Requested_Position ==
  DataServices_DID_D934_DID_D934_1_Requested_Position_ReadData_sys : Subsystem

== system cddDcmApp/DataServices_DID_D934_DID_D934_2_Actual_Position ==
  DataServices_DID_D934_DID_D934_2_Actual_Position_ReadData_sys : Subsystem

== system cddDcmApp/DataServices_DID_D935_DID_D935_1_step_Range ==
  DataServices_DID_D935_DID_D935_1_step_Range_ReadData_sys : Subsystem
  DataServices_DID_D935_DID_D935_1_step_Range_WriteData_sys : Subsystem

== system cddDcmApp/DataServices_DID_D935_DID_D935_2_No_Of_Movements ==
  DataServices_DID_D935_DID_D935_2_No_Of_Movements_ReadData_sys : Subsystem
  DataServices_DID_D935_DID_D935_2_No_Of_Movements_WriteData_sys : Subsystem

== system cddDcmApp/DataServices_DID_D936_ComplexStructure_2 ==
  DataServices_DID_D936_ComplexStructure_2_ReadData_sys : Subsystem

== system cddDcmApp/DataServices_DID_D936_DID_D936_1_Requested_Position ==
  DataServices_DID_D936_DID_D936_1_Requested_Position_ReadData_sys : Subsystem

== system cddDcmApp/DataServices_DID_D936_DID_D936_2_Actual_Position ==
  DataServices_DID_D936_DID_D936_2_Actual_Position_ReadData_sys : Subsystem

== system cddDcmApp/DataServices_DID_D937_DID_D937_1_step_Range ==
  DataServices_DID_D937_DID_D937_1_step_Range_ReadData_sys : Subsystem
  DataServices_DID_D937_DID_D937_1_step_Range_WriteData_sys : Subsystem

== system cddDcmApp/DataServices_DID_D937_DID_D937_2_No_Of_Movements ==
  DataServices_DID_D937_DID_D937_2_No_Of_Movements_ReadData_sys : Subsystem
  DataServices_DID_D937_DID_D937_2_No_Of_Movements_WriteData_sys : Subsystem

== system cddDcmApp/DataServices_DID_D938_ComplexStructure_2 ==
  DataServices_DID_D938_ComplexStructure_2_ReadData_sys : Subsystem

== system cddDcmApp/DataServices_DID_D938_DID_D938_1_Requested_Position ==
  DataServices_DID_D938_DID_D938_1_Requested_Position_ReadData_sys : Subsystem

== system cddDcmApp/DataServices_DID_D938_DID_D938_2_Actual_Position ==
  DataServices_DID_D938_DID_D938_2_Actual_Position_ReadData_sys : Subsystem

== system cddDcmApp/DataServices_DID_D939_DID_D939_1_step_Range ==
  DataServices_DID_D939_DID_D939_1_step_Range_ReadData_sys : Subsystem
  DataServices_DID_D939_DID_D939_1_step_Range_WriteData_sys : Subsystem

== system cddDcmApp/DataServices_DID_D939_DID_D939_2_No_Of_Movements ==
  DataServices_DID_D939_DID_D939_2_No_Of_Movements_ReadData_sys : Subsystem
  DataServices_DID_D939_DID_D939_2_No_Of_Movements_WriteData_sys : Subsystem

== system cddDcmApp/DataServices_DID_D93A_ComplexStructure_2 ==
  DataServices_DID_D93A_ComplexStructure_2_ReadData_sys : Subsystem

== system cddDcmApp/DataServices_DID_D93A_DID_D93A_1_Requested_Position ==
  DataServices_DID_D93A_DID_D93A_1_Requested_Position_ReadData_sys : Subsystem

== system cddDcmApp/DataServices_DID_D93A_DID_D93A_2_Actual_Position ==
  DataServices_DID_D93A_DID_D93A_2_Actual_Position_ReadData_sys : Subsystem

== system cddDcmApp/DataServices_DID_D93B_DID_D93B_1_step_Range ==
  DataServices_DID_D93B_DID_D93B_1_step_Range_ReadData_sys : Subsystem
  DataServices_DID_D93B_DID_D93B_1_step_Range_WriteData_sys : Subsystem

== system cddDcmApp/DataServices_DID_D93B_DID_D93B_2_No_Of_Movements ==
  DataServices_DID_D93B_DID_D93B_2_No_Of_Movements_ReadData_sys : Subsystem
  DataServices_DID_D93B_DID_D93B_2_No_Of_Movements_WriteData_sys : Subsystem

== system cddDcmApp/DataServices_DID_D93C_ComplexStructure_2 ==
  DataServices_DID_D93C_ComplexStructure_2_ReadData_sys : Subsystem

== system cddDcmApp/DataServices_DID_D93C_DID_D93C_1_Requested_Position ==
  DataServices_DID_D93C_DID_D93C_1_Requested_Position_ReadData_sys : Subsystem

== system cddDcmApp/DataServices_DID_D93C_DID_D93C_2_Actual_Position ==
  DataServices_DID_D93C_DID_D93C_2_Actual_Position_ReadData_sys : Subsystem

== system cddDcmApp/DataServices_DID_D93D_DID_D93D_1_step_Range ==
  DataServices_DID_D93D_DID_D93D_1_step_Range_ReadData_sys : Subsystem
  DataServices_DID_D93D_DID_D93D_1_step_Range_WriteData_sys : Subsystem

== system cddDcmApp/DataServices_DID_D93D_DID_D93D_2_No_Of_Movements ==
  DataServices_DID_D93D_DID_D93D_2_No_Of_Movements_ReadData_sys : Subsystem
  DataServices_DID_D93D_DID_D93D_2_No_Of_Movements_WriteData_sys : Subsystem

== system cddDcmApp/DataServices_DID_D93E_ComplexStructure_2 ==
  DataServices_DID_D93E_ComplexStructure_2_ReadData_sys : Subsystem

== system cddDcmApp/DataServices_DID_D93E_DID_D93E_1_Requested_Position ==
  DataServices_DID_D93E_DID_D93E_1_Requested_Position_ReadData_sys : Subsystem

== system cddDcmApp/DataServices_DID_D93E_DID_D93E_2_Actual_Position ==
  DataServices_DID_D93E_DID_D93E_2_Actual_Position_ReadData_sys : Subsystem

== system cddDcmApp/DataServices_DID_D93F_DID_D93F_1_step_Range ==
  DataServices_DID_D93F_DID_D93F_1_step_Range_ReadData_sys : Subsystem
  DataServices_DID_D93F_DID_D93F_1_step_Range_WriteData_sys : Subsystem

== system cddDcmApp/DataServices_DID_D93F_DID_D93F_2_No_Of_Movements ==
  DataServices_DID_D93F_DID_D93F_2_No_Of_Movements_ReadData_sys : Subsystem
  DataServices_DID_D93F_DID_D93F_2_No_Of_Movements_WriteData_sys : Subsystem

== system cddDcmApp/DataServices_DID_D940_ComplexStructure_2 ==
  DataServices_DID_D940_ComplexStructure_2_ReadData_sys : Subsystem

== system cddDcmApp/DataServices_DID_D940_DID_D940_1_Requested_Position ==
  DataServices_DID_D940_DID_D940_1_Requested_Position_ReadData_sys : Subsystem

== system cddDcmApp/DataServices_DID_D940_DID_D940_2_Actual_Position ==
  DataServices_DID_D940_DID_D940_2_Actual_Position_ReadData_sys : Subsystem

== system cddDcmApp/DataServices_DID_D941_DID_D941_1_step_Range ==
  DataServices_DID_D941_DID_D941_1_step_Range_ReadData_sys : Subsystem
  DataServices_DID_D941_DID_D941_1_step_Range_WriteData_sys : Subsystem

== system cddDcmApp/DataServices_DID_D941_DID_D941_2_No_Of_Movements ==
  DataServices_DID_D941_DID_D941_2_No_Of_Movements_ReadData_sys : Subsystem
  DataServices_DID_D941_DID_D941_2_No_Of_Movements_WriteData_sys : Subsystem

== system cddDcmApp/DataServices_DID_D942_ComplexStructure_2 ==
  DataServices_DID_D942_ComplexStructure_2_ReadData_sys : Subsystem

== system cddDcmApp/DataServices_DID_D942_DID_D942_1_Requested_Position ==
  DataServices_DID_D942_DID_D942_1_Requested_Position_ReadData_sys : Subsystem

== system cddDcmApp/DataServices_DID_D942_DID_D942_2_Actual_Position ==
  DataServices_DID_D942_DID_D942_2_Actual_Position_ReadData_sys : Subsystem

== system cddDcmApp/DataServices_DID_D943_DID_D943_1_step_Range ==
  DataServices_DID_D943_DID_D943_1_step_Range_ReadData_sys : Subsystem
  DataServices_DID_D943_DID_D943_1_step_Range_WriteData_sys : Subsystem

== system cddDcmApp/DataServices_DID_D943_DID_D943_2_No_Of_Movements ==
  DataServices_DID_D943_DID_D943_2_No_Of_Movements_ReadData_sys : Subsystem
  DataServices_DID_D943_DID_D943_2_No_Of_Movements_WriteData_sys : Subsystem

== system cddDcmApp/DataServices_DID_D944_ComplexStructure_2 ==
  DataServices_DID_D944_ComplexStructure_2_ReadData_sys : Subsystem

== system cddDcmApp/DataServices_DID_D944_DID_D944_1_Requested_Position ==
  DataServices_DID_D944_DID_D944_1_Requested_Position_ReadData_sys : Subsystem

== system cddDcmApp/DataServices_DID_D944_DID_D944_2_Actual_Position ==
  DataServices_DID_D944_DID_D944_2_Actual_Position_ReadData_sys : Subsystem

== system cddDcmApp/DataServices_DID_D945_DID_D945_1_step_Range ==
  DataServices_DID_D945_DID_D945_1_step_Range_ReadData_sys : Subsystem
  DataServices_DID_D945_DID_D945_1_step_Range_WriteData_sys : Subsystem

== system cddDcmApp/DataServices_DID_D945_DID_D945_2_No_Of_Movements ==
  DataServices_DID_D945_DID_D945_2_No_Of_Movements_ReadData_sys : Subsystem
  DataServices_DID_D945_DID_D945_2_No_Of_Movements_WriteData_sys : Subsystem

== system cddDcmApp/DataServices_DID_D946_ComplexStructure_2 ==
  DataServices_DID_D946_ComplexStructure_2_ReadData_sys : Subsystem

== system cddDcmApp/DataServices_DID_D946_DID_D946_1_Requested_Position ==
  DataServices_DID_D946_DID_D946_1_Requested_Position_ReadData_sys : Subsystem

== system cddDcmApp/DataServices_DID_D946_DID_D946_2_Actual_Position ==
  DataServices_DID_D946_DID_D946_2_Actual_Position_ReadData_sys : Subsystem

== system cddDcmApp/DataServices_DID_D947_DID_D947_1_step_Range ==
  DataServices_DID_D947_DID_D947_1_step_Range_ReadData_sys : Subsystem
  DataServices_DID_D947_DID_D947_1_step_Range_WriteData_sys : Subsystem

== system cddDcmApp/DataServices_DID_D947_DID_D947_2_No_Of_Movements ==
  DataServices_DID_D947_DID_D947_2_No_Of_Movements_ReadData_sys : Subsystem
  DataServices_DID_D947_DID_D947_2_No_Of_Movements_WriteData_sys : Subsystem

== system cddDcmApp/DataServices_DID_D948_ComplexStructure_2 ==
  DataServices_DID_D948_ComplexStructure_2_ReadData_sys : Subsystem

== system cddDcmApp/DataServices_DID_D948_DID_D948_1_Requested_Position ==
  DataServices_DID_D948_DID_D948_1_Requested_Position_ReadData_sys : Subsystem

== system cddDcmApp/DataServices_DID_D948_DID_D948_2_Actual_Position ==
  DataServices_DID_D948_DID_D948_2_Actual_Position_ReadData_sys : Subsystem

== system cddDcmApp/DataServices_DID_D94C_THM_SW_Version_DID_D94C_1_THM_SW_Version ==
  DataServices_DID_D94C_THM_SW_Version_DID_D94C_1_THM_SW_Version_ReadData_sys : Subsystem

== system cddDcmApp/DataServices_DID_D94C_THM_SW_Version_DID_D94C_2_Calibration_Version ==
  DataServices_DID_D94C_THM_SW_Version_DID_D94C_2_Calibration_Version_ReadData_sys : Subsystem

== system cddDcmApp/DataServices_DID_D950_DID_D950_1_DC_motor_Maximum_Calibration_limit ==
  DataServices_DID_D950_DID_D950_1_DC_motor_Maximum_Calibration_limit_ReadData_sys : Subsystem
  DataServices_DID_D950_DID_D950_1_DC_motor_Maximum_Calibration_limit_WriteData_sys : Subsystem

== system cddDcmApp/DataServices_DID_D950_DID_D950_2_DC_motor_Actual_ignition_counter ==
  DataServices_DID_D950_DID_D950_2_DC_motor_Actual_ignition_counter_ReadData_sys : Subsystem
  DataServices_DID_D950_DID_D950_2_DC_motor_Actual_ignition_counter_WriteData_sys : Subsystem

== system cddDcmApp/DataServices_DID_D950_DID_D950_3_HFA_step_motor_Maximum_Calibration_limit ==
  DataServices_DID_D950_DID_D950_3_HFA_step_motor_Maximum_Calibration_limit_ReadData_sys : Subsystem
  DataServices_DID_D950_DID_D950_3_HFA_step_motor_Maximum_Calibration_limit_WriteData_sys : Subsystem

== system cddDcmApp/DataServices_DID_D950_DID_D950_4_HFA_step_motor_Actual_ignition_counter ==
  DataServices_DID_D950_DID_D950_4_HFA_step_motor_Actual_ignition_counter_ReadData_sys : Subsystem
  DataServices_DID_D950_DID_D950_4_HFA_step_motor_Actual_ignition_counter_WriteData_sys : Subsystem

== system cddDcmApp/DataServices_DID_D950_DID_D950_5_VGA_step_Maximum_Calibration_limit ==
  DataServices_DID_D950_DID_D950_5_VGA_step_Maximum_Calibration_limit_ReadData_sys : Subsystem
  DataServices_DID_D950_DID_D950_5_VGA_step_Maximum_Calibration_limit_WriteData_sys : Subsystem

== system cddDcmApp/DataServices_DID_D950_DID_D950_6_VGA_step_Actual_ignition_counter ==
  DataServices_DID_D950_DID_D950_6_VGA_step_Actual_ignition_counter_ReadData_sys : Subsystem
  DataServices_DID_D950_DID_D950_6_VGA_step_Actual_ignition_counter_WriteData_sys : Subsystem

== system cddDcmApp/DataServices_DID_D951_DID_D951_1_AQS_HS_Smart_Control ==
  DataServices_DID_D951_DID_D951_1_AQS_HS_Smart_Control_ReadData_sys : Subsystem

== system cddDcmApp/DataServices_DID_D951_DID_D951_2_VBAT_short_circuit_detection ==
  DataServices_DID_D951_DID_D951_2_VBAT_short_circuit_detection_ReadData_sys : Subsystem

== system cddDcmApp/DataServices_DID_D951_DID_D951_3_Rear_ETXV_LS_Smart_Control ==
  DataServices_DID_D951_DID_D951_3_Rear_ETXV_LS_Smart_Control_ReadData_sys : Subsystem

== system cddDcmApp/DataServices_DID_D951_DID_D951_4_LIN_Power_Supply ==
  DataServices_DID_D951_DID_D951_4_LIN_Power_Supply_ReadData_sys : Subsystem

== system cddDcmApp/DataServices_DID_D951_DID_D951_5_Rear_PTC_LS_Smart_Control ==
  DataServices_DID_D951_DID_D951_5_Rear_PTC_LS_Smart_Control_ReadData_sys : Subsystem

== system cddDcmApp/DataServices_DID_D951_DID_D951_6_VGARHFA_motor_power_switch ==
  DataServices_DID_D951_DID_D951_6_VGARHFA_motor_power_switch_ReadData_sys : Subsystem

== system cddDcmApp/DataServices_DID_D957_DID_D957_MistRiskLvl ==
  DataServices_DID_D957_DID_D957_MistRiskLvl_ReadData_sys : Subsystem

== system cddDcmApp/DataServices_DID_D958_DID_D958_1_step_Range ==
  DataServices_DID_D958_DID_D958_1_step_Range_ReadData_sys : Subsystem
  DataServices_DID_D958_DID_D958_1_step_Range_WriteData_sys : Subsystem

== system cddDcmApp/DataServices_DID_D958_DID_D958_2_No_Of_Movements ==
  DataServices_DID_D958_DID_D958_2_No_Of_Movements_ReadData_sys : Subsystem
  DataServices_DID_D958_DID_D958_2_No_Of_Movements_WriteData_sys : Subsystem

== system cddDcmApp/DataServices_DID_D959_ComplexStructure_2 ==
  DataServices_DID_D959_ComplexStructure_2_ReadData_sys : Subsystem

== system cddDcmApp/DataServices_DID_D959_DID_D959_1_Requested_Position ==
  DataServices_DID_D959_DID_D959_1_Requested_Position_ReadData_sys : Subsystem

== system cddDcmApp/DataServices_DID_D959_DID_D959_2_Actual_Position ==
  DataServices_DID_D959_DID_D959_2_Actual_Position_ReadData_sys : Subsystem

== system cddDcmApp/DataServices_DID_D95A_DID_D95A_1_step_Range ==
  DataServices_DID_D95A_DID_D95A_1_step_Range_ReadData_sys : Subsystem
  DataServices_DID_D95A_DID_D95A_1_step_Range_WriteData_sys : Subsystem

== system cddDcmApp/DataServices_DID_D95A_DID_D95A_2_No_Of_Movements ==
  DataServices_DID_D95A_DID_D95A_2_No_Of_Movements_ReadData_sys : Subsystem
  DataServices_DID_D95A_DID_D95A_2_No_Of_Movements_WriteData_sys : Subsystem

== system cddDcmApp/DataServices_DID_D95B_ComplexStructure_2 ==
  DataServices_DID_D95B_ComplexStructure_2_ReadData_sys : Subsystem

== system cddDcmApp/DataServices_DID_D95B_DID_D95B_1_Requested_Position ==
  DataServices_DID_D95B_DID_D95B_1_Requested_Position_ReadData_sys : Subsystem

== system cddDcmApp/DataServices_DID_D95B_DID_D95B_2_Actual_Position ==
  DataServices_DID_D95B_DID_D95B_2_Actual_Position_ReadData_sys : Subsystem

== system cddDcmApp/DataServices_DID_D95C_DID_D95C_1_step_Range ==
  DataServices_DID_D95C_DID_D95C_1_step_Range_ReadData_sys : Subsystem
  DataServices_DID_D95C_DID_D95C_1_step_Range_WriteData_sys : Subsystem

== system cddDcmApp/DataServices_DID_D95C_DID_D95C_2_No_Of_Movements ==
  DataServices_DID_D95C_DID_D95C_2_No_Of_Movements_ReadData_sys : Subsystem
  DataServices_DID_D95C_DID_D95C_2_No_Of_Movements_WriteData_sys : Subsystem

== system cddDcmApp/DataServices_DID_D95D_ComplexStructure_2 ==
  DataServices_DID_D95D_ComplexStructure_2_ReadData_sys : Subsystem

== system cddDcmApp/DataServices_DID_D95D_DID_D95D_1_Requested_Position ==
  DataServices_DID_D95D_DID_D95D_1_Requested_Position_ReadData_sys : Subsystem

== system cddDcmApp/DataServices_DID_D95D_DID_D95D_2_Actual_Position ==
  DataServices_DID_D95D_DID_D95D_2_Actual_Position_ReadData_sys : Subsystem

== system cddDcmApp/DataServices_DID_D95E_DID_D95E_1_step_Range ==
  DataServices_DID_D95E_DID_D95E_1_step_Range_ReadData_sys : Subsystem
  DataServices_DID_D95E_DID_D95E_1_step_Range_WriteData_sys : Subsystem

== system cddDcmApp/DataServices_DID_D95E_DID_D95E_2_No_Of_Movements ==
  DataServices_DID_D95E_DID_D95E_2_No_Of_Movements_ReadData_sys : Subsystem
  DataServices_DID_D95E_DID_D95E_2_No_Of_Movements_WriteData_sys : Subsystem

== system cddDcmApp/DataServices_DID_D95F_ComplexStructure_2 ==
  DataServices_DID_D95F_ComplexStructure_2_ReadData_sys : Subsystem

== system cddDcmApp/DataServices_DID_D95F_DID_D95F_1_Requested_Position ==
  DataServices_DID_D95F_DID_D95F_1_Requested_Position_ReadData_sys : Subsystem

== system cddDcmApp/DataServices_DID_D95F_DID_D95F_2_Actual_Position ==
  DataServices_DID_D95F_DID_D95F_2_Actual_Position_ReadData_sys : Subsystem

== system cddDcmApp/DataServices_DID_D960_DID_D960_1_step_Range ==
  DataServices_DID_D960_DID_D960_1_step_Range_ReadData_sys : Subsystem
  DataServices_DID_D960_DID_D960_1_step_Range_WriteData_sys : Subsystem

== system cddDcmApp/DataServices_DID_D960_DID_D960_2_No_Of_Movements ==
  DataServices_DID_D960_DID_D960_2_No_Of_Movements_ReadData_sys : Subsystem
  DataServices_DID_D960_DID_D960_2_No_Of_Movements_WriteData_sys : Subsystem

== system cddDcmApp/DataServices_DID_D961_ComplexStructure_2 ==
  DataServices_DID_D961_ComplexStructure_2_ReadData_sys : Subsystem

== system cddDcmApp/DataServices_DID_D961_DID_D961_1_Requested_Position ==
  DataServices_DID_D961_DID_D961_1_Requested_Position_ReadData_sys : Subsystem

== system cddDcmApp/DataServices_DID_D961_DID_D961_2_Actual_Position ==
  DataServices_DID_D961_DID_D961_2_Actual_Position_ReadData_sys : Subsystem

== system cddDcmApp/DataServices_DID_D962_DID_D962_1_step_Range ==
  DataServices_DID_D962_DID_D962_1_step_Range_ReadData_sys : Subsystem
  DataServices_DID_D962_DID_D962_1_step_Range_WriteData_sys : Subsystem
